simulink model "LKA_Real_Life_Reference_cs"  (Simulink 7.6)
settings: Solver=ode5  SolverMode=SingleTasking  StartTime=0.0  StopTime=inf  FixedStep=1/100  RelTol=1e-3
model workspace (MAT data in the model file):
  mbox_table : struct (value not decoded)
  model_closed = 0
  sim_status = 0
PreLoadFcn:
  | check_NUTS_defined;
  | 
  | assignin('base',['model_closed_' bdroot],false);
  | 
  | if exist('LDW_mask_sfun.mexw64')==3
  | delete LDW_mask_sfun.mexw64;
  | end
  | 
  | if exist('LDW_mask_sfun.mexw32') ==3
  | delete LDW_mask_sfun.mexw32;
  | end
  | 
  | switch version('-release')
  | case {'2010a', '2010b', '2011a'}
  | addpath('../includes_LKAS/includes_LKAS_2010a');
  | case {'2011b', '2012a', '2012b'}
  | addpath('../includes_LKAS/includes_LKAS_2011b');
  | otherwise
  | addpath('../includes_LKAS/includes_LKAS_2011b');
  | end
InitFcn:
  | PreScanExperimentInitFcn(bdroot)
StopFcn:
  | PreScanExperimentStopFcn(bdroot)
undefined symbols (not defined in the file): DiscIntNeverNeededParam, InitImageOutputDir, x_ini_VRML, y_ini_VRML, z_ini_VRML
libraries: PreScanSimulinkLibrary x84, simulink x28, PreScanUsefulBlocks x25, simulink_extras x23, dspsnks4 x2, simulink_need_slupdate x1
(part 1 of 33)
CloseFcn:
  | evalin('base',['clear model_closed_' bdroot]);
  | 
  | set(0, 'ShowHiddenHandles', 'on')
  | 
  | try
  |     close (PlotSpeed)
  | end
  | 
  | try
  |     close(findobj('Name','Filtered'))
  | end
  | 
  | try
  |     close(findobj('Name','Results'))
  | end
  | 
  | set(0, 'ShowHiddenHandles', 'off')
settings Model: Creator=PRESCAN  LastModifiedBy=buildbot
settings OptimizationCC: InitFltsAndDblsToZero=on
settings DebuggingCC: SaveWithParameterizedLinksMsg=none  SignalResolutionControl=TryResolveAllWithWarning  SolverPrmCheckMsg=none  MultiTaskDSMMsg=warning  MultiTaskCondExecSysMsg=none
settings SFSimCC: SimParseCustomCode=off
settings RTWCC: IncludeERTFirstTime=on
settings GRTTargetCC: TargetFcnLib=ansi_tfl_tmw.mat

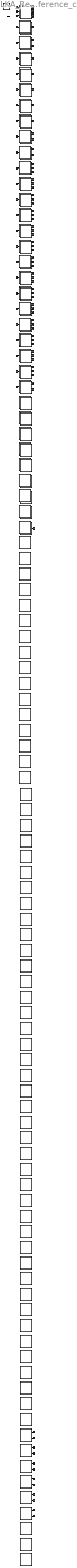
== system LKA_Real_Life_Reference_cs ==
  note: Demo Help
  note: Regenerate
  note: Record for Replay
  note: Open Useful Blocks
  note: PreScan Options
  note: Version:
  note: 7.4.0
  note: Author(s):
  note: N.A.
  note: Descriptive text:
  note: ID
      |
      | 1
      | 2
      | 483
      | 484
      | 485
      | 828
      | 830
      | 486
      | 838
      | 959
      | 992
      | 999
      | 1001
      | 1002
      | 1003
      | 1004
      | 1005
      | 1006
      | 1007
      | 1008
      | 1009
      | 1010
      | 1011
      | 1012
      | 1000
      | 639
      | 751
      | 757
      | 758
      | 759
      | 760
      | 761
      | 762
      | 763
      | 764
      | 765
      | 766
      | 767
      | 768
      | 769
      | 770
      | 771
      | 772
      | 773
      | 774
      | 775
      | 776
      | 777
      | 778
      | 779
      | 780
      | 781
      | 782
      | 783
      | 784
      | 785
      | 786
      | 787
      | 788
      | 789
      | 790
      | 791
      | 792
      | 793
      | 794
      | 795
      | 796
      | 797
      | 798
      | 799
      | 800
      | 801
      | 802
      | 803
      | 804
      | 805
      | 806
      | 807
      | 808
      | 809
      | 810
      | 811
      | 812
      | 813
      | 814
      | 815
      | 816
      | 817
      | 818
      | 819
      | 820
      | 115
      | 973
      | 985
      | 988
      | 993
      | 994
      | 1069
      | 1070
      | 1085
      | 1086
      | 1097
      | 1098
      | 1111
      | 1112
      | 1125
      | 1126
      | 1139
      | 1140
      | 1148
      | 1149
      | 1156
      | 1157
      | 1170
      | 1171
      | 1186
      | 1187
      | 1198
      | 1199
      | 1212
      | 1213
      | 1226
      | 1227
      | 1240
      | 1241
      | 1254
      | 1255
      | 1268
      | 1269
      | 1282
      | 1283
      | 1296
      | 1297
      | 1309
      | 1141
      | 1476
      | 1477
      | 1478
      | 1479
      | 1480
      | 1481
      | 1482
      | 1483
      | 1484
      | 1485
      | 1486
      | 1487
      | 1488
      | 1489
      | 1490
      | 1491
      | 1492
      | 1493
      | 1494
      | 1495
      | 1496
      | 1497
      | 1498
      | 1499
      | 1500
      | 1501
      | 1502
      | 1503
      | 1504
      | 1505
  note: OBU-Beacon ID
  note: TypeID
      |
      | 1
      | 5
      | 3
      | 3
      | 7
      | 3
      | 7
      | 3
      | 1
      | 1
      | 1
      | 1
      | 1
      | 1
      | 1
      | 1
      | 1
      | 1
      | 1
      | 1
      | 1
      | 1
      | 1
      | 1
      | 3
      | 15
      | 15
      | 15
      | 15
      | 15
      | 15
      | 15
      | 15
      | 15
      | 15
      | 15
      | 15
      | 15
      | 15
      | 15
      | 15
      | 15
      | 15
      | 15
      | 15
      | 15
      | 15
      | 15
      | 15
      | 15
      | 15
      | 15
      | 15
      | 15
      | 15
      | 15
      | 15
      | 15
      | 15
      | 15
      | 15
      | 15
      | 15
      | 15
      | 15
      | 15
      | 15
      | 15
      | 15
      | 15
      | 15
      | 15
      | 15
      | 15
      | 15
      | 15
      | 15
      | 15
      | 15
      | 15
      | 15
      | 15
      | 15
      | 15
      | 15
      | 15
      | 15
      | 15
      | 15
      | 15
      | 15
      | 15
      | 15
      | 15
      | 15
      | 15
      | 15
      | 15
      | 15
      | 15
      | 15
      | 15
      | 15
      | 15
      | 15
      | 15
      | 15
      | 15
      | 15
      | 15
      | 15
      | 15
      | 15
      | 15
      | 15
      | 15
      | 15
      | 15
      | 15
      | 15
      | 15
      | 15
      | 15
      | 15
      | 15
      | 15
      | 15
      | 15
      | 15
      | 15
      | 15
      | 15
      | 15
      | 15
      | 15
      | 15
      | 15
      | 15
      | 15
      | 15
      | 15
      | 15
      | 15
      | 15
      | 15
      | 15
      | 15
      | 15
      | 15
      | 15
      | 15
      | 15
      | 15
      | 15
      | 15
      | 15
      | 15
      | 15
      | 15
      | 15
      | 15
      | 15
      | 15
      | 15
      | 15
  note: TypeTag
      |
      | Car
      | Cal element
      | Truck\Bus
      | Truck\Bus
      | Unknown
      | Truck\Bus
      | Unknown
      | Truck\Bus
      | Car
      | Car
      | Car
      | Car
      | Car
      | Car
      | Car
      | Car
      | Car
      | Car
      | Car
      | Car
      | Car
      | Car
      | Car
      | Car
      | Truck\Bus
      | Anim traffic sign
      | Anim traffic sign
      | Anim traffic sign
      | Anim traffic sign
      | Anim traffic sign
      | Anim traffic sign
      | Anim traffic sign
      | Anim traffic sign
      | Anim traffic sign
      | Anim traffic sign
      | Anim traffic sign
      | Anim traffic sign
      | Anim traffic sign
      | Anim traffic sign
      | Anim traffic sign
      | Anim traffic sign
      | Anim traffic sign
      | Anim traffic sign
      | Anim traffic sign
      | Anim traffic sign
      | Anim traffic sign
      | Anim traffic sign
      | Anim traffic sign
      | Anim traffic sign
      | Anim traffic sign
      | Anim traffic sign
      | Anim traffic sign
      | Anim traffic sign
      | Anim traffic sign
      | Anim traffic sign
      | Anim traffic sign
      | Anim traffic sign
      | Anim traffic sign
      | Anim traffic sign
      | Anim traffic sign
      | Anim traffic sign
      | Anim traffic sign
      | Anim traffic sign
      | Anim traffic sign
      | Anim traffic sign
      | Anim traffic sign
      | Anim traffic sign
      | Anim traffic sign
      | Anim traffic sign
      | Anim traffic sign
      | Anim traffic sign
      | Anim traffic sign
      | Anim traffic sign
      | Anim traffic sign
      | Anim traffic sign
      | Anim traffic sign
      | Anim traffic sign
      | Anim traffic sign
      | Anim traffic sign
      | Anim traffic sign
      | Anim traffic sign
      | Anim traffic sign
      | Anim traffic sign
      | Anim traffic sign
      | Anim traffic sign
      | Anim traffic sign
      | Anim traffic sign
      | Anim traffic sign
      | Anim traffic sign
      | Anim traffic sign
      | Anim traffic sign
      | Anim traffic sign
      | Anim traffic sign
      | Anim traffic sign
      | Anim traffic sign
      | Anim traffic sign
      | Anim traffic sign
      | Anim traffic sign
      | Anim traffic sign
      | Anim traffic sign
      | Anim traffic sign
      | Anim traffic sign
      | Anim traffic sign
      | Anim traffic sign
      | Anim traffic sign
      | Anim traffic sign
      | Anim traffic sign
      | Anim traffic sign
      | Anim traffic sign
      | Anim traffic sign
      | Anim traffic sign
      | Anim traffic sign
      | Anim traffic sign
      | Anim traffic sign
      | Anim traffic sign
      | Anim traffic sign
      | Anim traffic sign
      | Anim traffic sign
      | Anim traffic sign
      | Anim traffic sign
      | Anim traffic sign
      | Anim traffic sign
      | Anim traffic sign
      | Anim traffic sign
      | Anim traffic sign
      | Anim traffic sign
      | Anim traffic sign
      | Anim traffic sign
      | Anim traffic sign
      | Anim traffic sign
      | Anim traffic sign
      | Anim traffic sign
      | Anim traffic sign
      | Anim traffic sign
      | Anim traffic sign
      | Anim traffic sign
      | Anim traffic sign
      | Anim traffic sign
      | Anim traffic sign
      | Anim traffic sign
      | Anim traffic sign
      | Anim traffic sign
      | Anim traffic sign
      | Anim traffic sign
      | Anim traffic sign
      | Anim traffic sign
      | Anim traffic sign
      | Anim traffic sign
      | Anim traffic sign
      | Anim traffic sign
      | Anim traffic sign
      | Anim traffic sign
      | Anim traffic sign
      | Anim traffic sign
      | Anim traffic sign
      | Anim traffic sign
      | Anim traffic sign
      | Anim traffic sign
      | Anim traffic sign
      | Anim traffic sign
      | Anim traffic sign
      | Anim traffic sign
      | Anim traffic sign
      | Anim traffic sign
      | Anim traffic sign
  note: SD
      |
      | Y
      | Y
      | Y
      | Y
      | Y
      | Y
      | Y
      | Y
      | Y
      | Y
      | Y
      | Y
      | Y
      | Y
      | Y
      | Y
      | Y
      | Y
      | Y
      | Y
      | Y
      | Y
      | Y
      | Y
      | Y
      | N
      | N
      | N
      | N
      | N
      | N
      | N
      | N
      | N
      | N
      | N
      | N
      | N
      | N
      | N
      | N
      | N
      | N
      | N
      | N
      | N
      | N
      | N
      | N
      | N
      | N
      | N
      | N
      | N
      | N
      | N
      | N
      | N
      | N
      | N
      | N
      | N
      | N
      | N
      | N
      | N
      | N
      | N
      | N
      | N
      | N
      | N
      | N
      | N
      | N
      | N
      | N
      | N
      | N
      | N
      | N
      | N
      | N
      | N
      | N
      | N
      | N
      | N
      | N
      | N
      | N
      | Y
      | Y
      | Y
      | Y
      | Y
      | Y
      | N
      | N
      | N
      | N
      | N
      | N
      | N
      | N
      | N
      | N
      | N
      | N
      | N
      | N
      | N
      | N
      | N
      | N
      | N
      | N
      | N
      | N
      | N
      | N
      | N
      | N
      | N
      | N
      | N
      | N
      | N
      | N
      | N
      | N
      | N
      | N
      | Y
      | Y
      | Y
      | Y
      | Y
      | Y
      | Y
      | Y
      | Y
      | Y
      | Y
      | Y
      | Y
      | Y
      | Y
      | Y
      | Y
      | Y
      | Y
      | Y
      | Y
      | Y
      | Y
      | Y
      | Y
      | Y
      | Y
      | Y
      | Y
      | Y
      | Y
      | Y
  note: CD
      |
      | N
      | N
      | N
      | N
      | N
      | N
      | N
      | N
      | N
      | N
      | N
      | N
      | N
      | N
      | N
      | N
      | N
      | N
      | N
      | N
      | N
      | N
      | N
      | N
      | N
      | N
      | N
      | N
      | N
      | N
      | N
      | N
      | N
      | N
      | N
      | N
      | N
      | N
      | N
      | N
      | N
      | N
      | N
      | N
      | N
      | N
      | N
      | N
      | N
      | N
      | N
      | N
      | N
      | N
      | N
      | N
      | N
      | N
      | N
      | N
      | N
      | N
      | N
      | N
      | N
      | N
      | N
      | N
      | N
      | N
      | N
      | N
      | N
      | N
      | N
      | N
      | N
      | N
      | N
      | N
      | N
      | N
      | N
      | N
      | N
      | N
      | N
      | N
      | N
      | N
      | N
      | N
      | N
      | N
      | N
      | N
      | N
      | N
      | N
      | N
      | N
      | N
      | N
      | N
      | N
      | N
      | N
      | N
      | N
      | N
      | N
      | N
      | N
      | N
      | N
      | N
      | N
      | N
      | N
      | N
      | N
      | N
      | N
      | N
      | N
      | N
      | N
      | N
      | N
      | N
      | N
      | N
      | N
      | N
      | N
      | N
      | N
      | N
      | N
      | N
      | N
      | N
      | N
      | N
      | N
      | N
      | N
      | N
      | N
      | N
      | N
      | N
      | N
      | N
      | N
      | N
      | N
      | N
      | N
      | N
      | N
      | N
      | N
      | N
      | N
  note: Name
      |
      | Audi_A8_1
      | Sphere_1
      | Nissan_Cabstar_1
      | Actros_1860_1
      | ContainerTrailer_DoubleAxle_1
      | Actros_1860_2
      | ContainerTrailer_DoubleAxle_2
      | Actros_2541_1
      | Toyota_Previa_1
      | Mazda_RX8_1
      | Mazda_RX8_2
      | Ford_Fiesta_1
      | BMW_Z3_1
      | Audi_A8_2
      | Citroen_C3_1
      | Citroen_C3_2
      | Toyota_Yaris_1
      | Fiat_Bravo_1
      | Citroen_C3_3
      | Toyota_Previa_2
      | Mazda_RX8_3
      | Fiat_Bravo_2
      | BMW_Z3_2
      | Citroen_C3_4
      | Actros_2541_2
      | Lightpost_1
      | Lightpost_2
      | Lightpost_3
      | Lightpost_4
      | Lightpost_5
      | Lightpost_6
      | Lightpost_7
      | Lightpost_8
      | Lightpost_9
      | Lightpost_10
      | Lightpost_11
      | Lightpost_12
      | Lightpost_13
      | Lightpost_14
      | Lightpost_15
      | Lightpost_16
      | Lightpost_17
      | Lightpost_18
      | Lightpost_19
      | Lightpost_20
      | Lightpost_21
      | Lightpost_22
      | Lightpost_23
      | Lightpost_24
      | Lightpost_25
      | Lightpost_26
      | Lightpost_27
      | Lightpost_28
      | Lightpost_29
      | Lightpost_30
      | Lightpost_31
      | Lightpost_32
      | Lightpost_33
      | Lightpost_34
      | Lightpost_35
      | Lightpost_36
      | Lightpost_37
      | Lightpost_38
      | Lightpost_39
      | Lightpost_40
      | Lightpost_41
      | Lightpost_42
      | Lightpost_43
      | Lightpost_44
      | Lightpost_45
      | Lightpost_46
      | Lightpost_47
      | Lightpost_48
      | Lightpost_49
      | Lightpost_50
      | Lightpost_51
      | Lightpost_52
      | Lightpost_53
      | Lightpost_54
      | Lightpost_55
      | Lightpost_56
      | Lightpost_57
      | Lightpost_58
      | Lightpost_59
      | Lightpost_60
      | Lightpost_61
      | Lightpost_62
      | Lightpost_63
      | Lightpost_64
      | Lightpost_65
      | Lightpost_66
      | HighwayOverhang_1
      | HighwayOverhang_2
      | HighwayOverhang_3
      | HighwayOverhang_4
      | HighwayOverhang_5
      | HighwayOverhang_6
      | Lightpost_67
      | Lightpost_68
      | Lightpost_71
      | Lightpost_72
      | Lightpost_69
      | Lightpost_70
      | Lightpost_73
      | Lightpost_74
      | Lightpost_75
      | Lightpost_76
      | Lightpost_77
      | Lightpost_78
      | Lightpost_79
      | Lightpost_80
      | Lightpost_81
      | Lightpost_82
      | Lightpost_83
      | Lightpost_84
      | Lightpost_87
      | Lightpost_88
      | Lightpost_85
      | Lightpost_86
      | Lightpost_89
      | Lightpost_90
      | Lightpost_91
      | Lightpost_92
      | Lightpost_93
      | Lightpost_94
      | Lightpost_95
      | Lightpost_96
      | Lightpost_97
      | Lightpost_98
      | Lightpost_99
      | Lightpost_100
      | Lightpost_101
      | Lightpost_102
      | InformationBoard_1
      | InformationBoard_2
      | InformationBoard_3
      | InformationBoard_4
      | InformationBoard_5
      | InformationBoard_6
      | InformationBoard_7
      | InformationBoard_8
      | InformationBoard_9
      | InformationBoard_10
      | InformationBoard_11
      | InformationBoard_12
      | InformationBoard_13
      | InformationBoard_14
      | InformationBoard_15
      | InformationBoard_16
      | InformationBoard_17
      | InformationBoard_18
      | InformationBoard_19
      | InformationBoard_20
      | InformationBoard_21
      | InformationBoard_22
      | InformationBoard_23
      | InformationBoard_24
      | InformationBoard_25
      | InformationBoard_26
      | InformationBoard_27
      | InformationBoard_28
      | InformationBoard_29
      | InformationBoard_30
      | InformationBoard_31
      | HighwayOverhang_7
  note: Num ID OBU-Beacon ID Type ID Type Tag SD CD Name
  Actros_1860_1 : Subsystem  Tag=WorldObjectSubSystem  InitFcn=UniqueId = '1580';
      mask Display: image(imread('Actros_1860.png'));color('white');text(0,0,' ID=484\n')
  Actros_1860_2 : Subsystem  Tag=WorldObjectSubSystem  InitFcn=UniqueId = '1584';
      mask Display: image(imread('Actros_1860.png'));color('white');text(0,0,' ID=828\n')
  Actros_2541_1 : Subsystem  Tag=WorldObjectSubSystem  InitFcn=UniqueId = '1588';
      mask Display: image(imread('Actros_2541.png'));color('white');text(0,0,' ID=486\n')
  Actros_2541_2 : Subsystem  Tag=WorldObjectSubSystem  InitFcn=UniqueId = '2575';
      mask Display: image(imread('Actros_2541.png'));color('white');text(0,0,' ID=1000\n')
  Actuators : DataStoreMemory  Tag=Actuators  DataStoreName=SimCoreActuators  InitialValue=zeros(2639,1)
      settings: DeleteFcn=PreScanBlockDeleteFcn;
  Audi_A8_1 : Subsystem  Tag=WorldObjectSubSystem  InitFcn=UniqueId = '253';
      mask Display: image(imread('Audi_A8.jpg'));color('white');text(0,0,' ID=1\n')
  Audi_A8_2 : Subsystem  Tag=WorldObjectSubSystem  InitFcn=UniqueId = '1951';
      mask Display: image(imread('Audi_A8.jpg'));color('white');text(0,0,' ID=1002\n')
  BMW_Z3_1 : Subsystem  Tag=WorldObjectSubSystem  InitFcn=UniqueId = '1948';
      mask Display: image(imread('BMW_Z3.jpg'));color('white');text(0,0,' ID=1001\n')
  BMW_Z3_2 : Subsystem  Tag=WorldObjectSubSystem  InitFcn=UniqueId = '2024';
      mask Display: image(imread('BMW_Z3.jpg'));color('white');text(0,0,' ID=1011\n')
  CameraSensor_2 : Subsystem  Tag=CameraSensor  Priority=3
      settings: DeleteFcn=PreScanBlockDeleteFcn;
      mask Type: PreScan Source Block: Camera Sensor
      mask Description: The Camera Sensor provides a color or monochrome image.
      mask Help: eval('openHelp(457)')
      mask Display: image(imread('CameraSensor.png'))
  Citroen_C3_1 : Subsystem  Tag=WorldObjectSubSystem  InitFcn=UniqueId = '1955';
      mask Display: image(imread('Citroen_C3.jpg'));color('white');text(0,0,' ID=1003\n')
  Citroen_C3_2 : Subsystem  Tag=WorldObjectSubSystem  InitFcn=UniqueId = '1958';
      mask Display: image(imread('Citroen_C3.jpg'));color('white');text(0,0,' ID=1004\n')
  Citroen_C3_3 : Subsystem  Tag=WorldObjectSubSystem  InitFcn=UniqueId = '1967';
      mask Display: image(imread('Citroen_C3.jpg'));color('white');text(0,0,' ID=1007\n')
  Citroen_C3_4 : Subsystem  Tag=WorldObjectSubSystem  InitFcn=UniqueId = '2027';
      mask Display: image(imread('Citroen_C3.jpg'));color('white');text(0,0,' ID=1012\n')
  ContainerTrailer_DoubleAxle_1 : Subsystem  Tag=WorldObjectSubSystem  InitFcn=UniqueId = '1582';
      mask Display: image(imread('ContainerTrailer_DoubleAxle.jpg'));color('white');text(0,0,' ID=485\n')
  ContainerTrailer_DoubleAxle_2 : Subsystem  Tag=WorldObjectSubSystem  InitFcn=UniqueId = '1586';
      mask Display: image(imread('ContainerTrailer_DoubleAxle.jpg'));color('white');text(0,0,' ID=830\n')
  Fiat_Bravo_1 : Subsystem  Tag=WorldObjectSubSystem  InitFcn=UniqueId = '1964';
      mask Display: image(imread('Fiat_Bravo.jpg'));color('white');text(0,0,' ID=1006\n')
  Fiat_Bravo_2 : Subsystem  Tag=WorldObjectSubSystem  InitFcn=UniqueId = '2021';
      mask Display: image(imread('Fiat_Bravo.jpg'));color('white');text(0,0,' ID=1010\n')
  Ford_Fiesta_1 : Subsystem  Tag=WorldObjectSubSystem  InitFcn=UniqueId = '1934';
      mask Display: image(imread('Ford_Fiesta.jpg'));color('white');text(0,0,' ID=999\n')
  GetSensors : Subsystem  Tag=GetSensors  Priority=2  mask(PreScan Simulation Core Block){NrOfSensors=200, TimeStep=1/100}
      mask prompts: NrOfSensors "Number of sensor values"; TimeStep "Timestep [s]"
      mask help:
      | Please do not remove this block from the PreScan compilation sheet,
      | as it contains essential configuration parameters for a PreScan simulation.
      settings: DeleteFcn=PreScanBlockDeleteFcn;
  HighwayOverhang_1 : Subsystem  Tag=WorldObjectSubSystem  InitFcn=UniqueId = '1876';
      mask Display: image(imread('HighwayOverhang.jpg'));color('white');text(0,0,' ID=115\n')
  HighwayOverhang_2 : Subsystem  Tag=WorldObjectSubSystem  InitFcn=UniqueId = '1877';
      mask Display: image(imread('HighwayOverhang.jpg'));color('white');text(0,0,' ID=973\n')
  HighwayOverhang_3 : Subsystem  Tag=WorldObjectSubSystem  InitFcn=UniqueId = '1890';
      mask Display: image(imread('HighwayOverhang.jpg'));color('white');text(0,0,' ID=985\n')
  HighwayOverhang_4 : Subsystem  Tag=WorldObjectSubSystem  InitFcn=UniqueId = '1898';
      mask Display: image(imread('HighwayOverhang.jpg'));color('white');text(0,0,' ID=988\n')
  HighwayOverhang_5 : Subsystem  Tag=WorldObjectSubSystem  InitFcn=UniqueId = '1909';
      mask Display: image(imread('HighwayOverhang.jpg'));color('white');text(0,0,' ID=993\n')
  HighwayOverhang_6 : Subsystem  Tag=WorldObjectSubSystem  InitFcn=UniqueId = '1914';
      mask Display: image(imread('HighwayOverhang.jpg'));color('white');text(0,0,' ID=994\n')
  HighwayOverhang_7 : Subsystem  Tag=WorldObjectSubSystem  InitFcn=UniqueId = '2612';
      mask Display: image(imread('HighwayOverhang.jpg'));color('white');text(0,0,' ID=1505\n')
  LaneMarkerSensor_1 : Subsystem  Tag=LaneMarkerSensor  Priority=3
      settings: DeleteFcn=PreScanBlockDeleteFcn;
      mask Type: PreScan Source Block: Lane Marker Sensor
      mask Description: The Lane Marker Sensor provides information relating the sensor to the lane markers on the road.
      mask Help: eval('openHelp(457)')
      mask Display: image(imread('LaneMarkerSensorSL.png'))
  Lightpost_1 : Subsystem  Tag=WorldObjectSubSystem  InitFcn=UniqueId = '1495';
      mask Display: image(imread('Lightpost.jpg'));color('white');text(0,0,' ID=639\n')
  Lightpost_10 : Subsystem  Tag=WorldObjectSubSystem  InitFcn=UniqueId = '1504';
      mask Display: image(imread('Lightpost.jpg'));color('white');text(0,0,' ID=764\n')
  Lightpost_100 : Subsystem  Tag=WorldObjectSubSystem  InitFcn=UniqueId = '2375';
      mask Display: image(imread('Lightpost.jpg'));color('white');text(0,0,' ID=1283\n')
  Lightpost_101 : Subsystem  Tag=WorldObjectSubSystem  InitFcn=UniqueId = '2390';
      mask Display: image(imread('Lightpost.jpg'));color('white');text(0,0,' ID=1296\n')
  Lightpost_102 : Subsystem  Tag=WorldObjectSubSystem  InitFcn=UniqueId = '2391';
      mask Display: image(imread('Lightpost.jpg'));color('white');text(0,0,' ID=1297\n')
  Lightpost_11 : Subsystem  Tag=WorldObjectSubSystem  InitFcn=UniqueId = '1505';
      mask Display: image(imread('Lightpost.jpg'));color('white');text(0,0,' ID=765\n')
  Lightpost_12 : Subsystem  Tag=WorldObjectSubSystem  InitFcn=UniqueId = '1506';
      mask Display: image(imread('Lightpost.jpg'));color('white');text(0,0,' ID=766\n')
  Lightpost_13 : Subsystem  Tag=WorldObjectSubSystem  InitFcn=UniqueId = '1507';
      mask Display: image(imread('Lightpost.jpg'));color('white');text(0,0,' ID=767\n')
  Lightpost_14 : Subsystem  Tag=WorldObjectSubSystem  InitFcn=UniqueId = '1508';
      mask Display: image(imread('Lightpost.jpg'));color('white');text(0,0,' ID=768\n')
  Lightpost_15 : Subsystem  Tag=WorldObjectSubSystem  InitFcn=UniqueId = '1509';
      mask Display: image(imread('Lightpost.jpg'));color('white');text(0,0,' ID=769\n')
  Lightpost_16 : Subsystem  Tag=WorldObjectSubSystem  InitFcn=UniqueId = '1510';
      mask Display: image(imread('Lightpost.jpg'));color('white');text(0,0,' ID=770\n')
  Lightpost_17 : Subsystem  Tag=WorldObjectSubSystem  InitFcn=UniqueId = '1511';
      mask Display: image(imread('Lightpost.jpg'));color('white');text(0,0,' ID=771\n')
  Lightpost_18 : Subsystem  Tag=WorldObjectSubSystem  InitFcn=UniqueId = '1512';
      mask Display: image(imread('Lightpost.jpg'));color('white');text(0,0,' ID=772\n')
  Lightpost_19 : Subsystem  Tag=WorldObjectSubSystem  InitFcn=UniqueId = '1513';
      mask Display: image(imread('Lightpost.jpg'));color('white');text(0,0,' ID=773\n')
  Lightpost_2 : Subsystem  Tag=WorldObjectSubSystem  InitFcn=UniqueId = '1496';
      mask Display: image(imread('Lightpost.jpg'));color('white');text(0,0,' ID=751\n')
  Lightpost_20 : Subsystem  Tag=WorldObjectSubSystem  InitFcn=UniqueId = '1514';
      mask Display: image(imread('Lightpost.jpg'));color('white');text(0,0,' ID=774\n')
  Lightpost_21 : Subsystem  Tag=WorldObjectSubSystem  InitFcn=UniqueId = '1515';
      mask Display: image(imread('Lightpost.jpg'));color('white');text(0,0,' ID=775\n')
  Lightpost_22 : Subsystem  Tag=WorldObjectSubSystem  InitFcn=UniqueId = '1516';
      mask Display: image(imread('Lightpost.jpg'));color('white');text(0,0,' ID=776\n')
  Lightpost_23 : Subsystem  Tag=WorldObjectSubSystem  InitFcn=UniqueId = '1517';
      mask Display: image(imread('Lightpost.jpg'));color('white');text(0,0,' ID=777\n')
  Lightpost_24 : Subsystem  Tag=WorldObjectSubSystem  InitFcn=UniqueId = '1518';
      mask Display: image(imread('Lightpost.jpg'));color('white');text(0,0,' ID=778\n')
  Lightpost_25 : Subsystem  Tag=WorldObjectSubSystem  InitFcn=UniqueId = '1519';
      mask Display: image(imread('Lightpost.jpg'));color('white');text(0,0,' ID=779\n')
  Lightpost_26 : Subsystem  Tag=WorldObjectSubSystem  InitFcn=UniqueId = '1520';
      mask Display: image(imread('Lightpost.jpg'));color('white');text(0,0,' ID=780\n')
  Lightpost_27 : Subsystem  Tag=WorldObjectSubSystem  InitFcn=UniqueId = '1521';
      mask Display: image(imread('Lightpost.jpg'));color('white');text(0,0,' ID=781\n')
  Lightpost_28 : Subsystem  Tag=WorldObjectSubSystem  InitFcn=UniqueId = '1522';
      mask Display: image(imread('Lightpost.jpg'));color('white');text(0,0,' ID=782\n')
  Lightpost_29 : Subsystem  Tag=WorldObjectSubSystem  InitFcn=UniqueId = '1523';
      mask Display: image(imread('Lightpost.jpg'));color('white');text(0,0,' ID=783\n')
  Lightpost_3 : Subsystem  Tag=WorldObjectSubSystem  InitFcn=UniqueId = '1497';
      mask Display: image(imread('Lightpost.jpg'));color('white');text(0,0,' ID=757\n')
  Lightpost_30 : Subsystem  Tag=WorldObjectSubSystem  InitFcn=UniqueId = '1524';
      mask Display: image(imread('Lightpost.jpg'));color('white');text(0,0,' ID=784\n')
  Lightpost_31 : Subsystem  Tag=WorldObjectSubSystem  InitFcn=UniqueId = '1525';
      mask Display: image(imread('Lightpost.jpg'));color('white');text(0,0,' ID=785\n')
  Lightpost_32 : Subsystem  Tag=WorldObjectSubSystem  InitFcn=UniqueId = '1526';
      mask Display: image(imread('Lightpost.jpg'));color('white');text(0,0,' ID=786\n')
  Lightpost_33 : Subsystem  Tag=WorldObjectSubSystem  InitFcn=UniqueId = '1527';
      mask Display: image(imread('Lightpost.jpg'));color('white');text(0,0,' ID=787\n')
  Lightpost_34 : Subsystem  Tag=WorldObjectSubSystem  InitFcn=UniqueId = '1528';
      mask Display: image(imread('Lightpost.jpg'));color('white');text(0,0,' ID=788\n')
  Lightpost_35 : Subsystem  Tag=WorldObjectSubSystem  InitFcn=UniqueId = '1529';
      mask Display: image(imread('Lightpost.jpg'));color('white');text(0,0,' ID=789\n')
  Lightpost_36 : Subsystem  Tag=WorldObjectSubSystem  InitFcn=UniqueId = '1530';
      mask Display: image(imread('Lightpost.jpg'));color('white');text(0,0,' ID=790\n')
  Lightpost_37 : Subsystem  Tag=WorldObjectSubSystem  InitFcn=UniqueId = '1531';
      mask Display: image(imread('Lightpost.jpg'));color('white');text(0,0,' ID=791\n')
  Lightpost_38 : Subsystem  Tag=WorldObjectSubSystem  InitFcn=UniqueId = '1532';
      mask Display: image(imread('Lightpost.jpg'));color('white');text(0,0,' ID=792\n')
  Lightpost_39 : Subsystem  Tag=WorldObjectSubSystem  InitFcn=UniqueId = '1533';
      mask Display: image(imread('Lightpost.jpg'));color('white');text(0,0,' ID=793\n')
  Lightpost_4 : Subsystem  Tag=WorldObjectSubSystem  InitFcn=UniqueId = '1498';
      mask Display: image(imread('Lightpost.jpg'));color('white');text(0,0,' ID=758\n')
  Lightpost_40 : Subsystem  Tag=WorldObjectSubSystem  InitFcn=UniqueId = '1534';
      mask Display: image(imread('Lightpost.jpg'));color('white');text(0,0,' ID=794\n')
  Lightpost_41 : Subsystem  Tag=WorldObjectSubSystem  InitFcn=UniqueId = '1535';
      mask Display: image(imread('Lightpost.jpg'));color('white');text(0,0,' ID=795\n')
  Lightpost_42 : Subsystem  Tag=WorldObjectSubSystem  InitFcn=UniqueId = '1536';
      mask Display: image(imread('Lightpost.jpg'));color('white');text(0,0,' ID=796\n')
  Lightpost_43 : Subsystem  Tag=WorldObjectSubSystem  InitFcn=UniqueId = '1537';
      mask Display: image(imread('Lightpost.jpg'));color('white');text(0,0,' ID=797\n')
  Lightpost_44 : Subsystem  Tag=WorldObjectSubSystem  InitFcn=UniqueId = '1538';
      mask Display: image(imread('Lightpost.jpg'));color('white');text(0,0,' ID=798\n')
  Lightpost_45 : Subsystem  Tag=WorldObjectSubSystem  InitFcn=UniqueId = '1539';
      mask Display: image(imread('Lightpost.jpg'));color('white');text(0,0,' ID=799\n')
  Lightpost_46 : Subsystem  Tag=WorldObjectSubSystem  InitFcn=UniqueId = '1540';
      mask Display: image(imread('Lightpost.jpg'));color('white');text(0,0,' ID=800\n')
  Lightpost_47 : Subsystem  Tag=WorldObjectSubSystem  InitFcn=UniqueId = '1541';
      mask Display: image(imread('Lightpost.jpg'));color('white');text(0,0,' ID=801\n')
  Lightpost_48 : Subsystem  Tag=WorldObjectSubSystem  InitFcn=UniqueId = '1542';
      mask Display: image(imread('Lightpost.jpg'));color('white');text(0,0,' ID=802\n')
  Lightpost_49 : Subsystem  Tag=WorldObjectSubSystem  InitFcn=UniqueId = '1543';
      mask Display: image(imread('Lightpost.jpg'));color('white');text(0,0,' ID=803\n')
  Lightpost_5 : Subsystem  Tag=WorldObjectSubSystem  InitFcn=UniqueId = '1499';
      mask Display: image(imread('Lightpost.jpg'));color('white');text(0,0,' ID=759\n')
  Lightpost_50 : Subsystem  Tag=WorldObjectSubSystem  InitFcn=UniqueId = '1544';
      mask Display: image(imread('Lightpost.jpg'));color('white');text(0,0,' ID=804\n')
  Lightpost_51 : Subsystem  Tag=WorldObjectSubSystem  InitFcn=UniqueId = '1545';
      mask Display: image(imread('Lightpost.jpg'));color('white');text(0,0,' ID=805\n')
  Lightpost_52 : Subsystem  Tag=WorldObjectSubSystem  InitFcn=UniqueId = '1546';
      mask Display: image(imread('Lightpost.jpg'));color('white');text(0,0,' ID=806\n')
  Lightpost_53 : Subsystem  Tag=WorldObjectSubSystem  InitFcn=UniqueId = '1547';
      mask Display: image(imread('Lightpost.jpg'));color('white');text(0,0,' ID=807\n')
  Lightpost_54 : Subsystem  Tag=WorldObjectSubSystem  InitFcn=UniqueId = '1548';
      mask Display: image(imread('Lightpost.jpg'));color('white');text(0,0,' ID=808\n')
  Lightpost_55 : Subsystem  Tag=WorldObjectSubSystem  InitFcn=UniqueId = '1549';
      mask Display: image(imread('Lightpost.jpg'));color('white');text(0,0,' ID=809\n')
  Lightpost_56 : Subsystem  Tag=WorldObjectSubSystem  InitFcn=UniqueId = '1550';
      mask Display: image(imread('Lightpost.jpg'));color('white');text(0,0,' ID=810\n')
  Lightpost_57 : Subsystem  Tag=WorldObjectSubSystem  InitFcn=UniqueId = '1551';
      mask Display: image(imread('Lightpost.jpg'));color('white');text(0,0,' ID=811\n')
  Lightpost_58 : Subsystem  Tag=WorldObjectSubSystem  InitFcn=UniqueId = '1552';
      mask Display: image(imread('Lightpost.jpg'));color('white');text(0,0,' ID=812\n')
  Lightpost_59 : Subsystem  Tag=WorldObjectSubSystem  InitFcn=UniqueId = '1553';
      mask Display: image(imread('Lightpost.jpg'));color('white');text(0,0,' ID=813\n')
  Lightpost_6 : Subsystem  Tag=WorldObjectSubSystem  InitFcn=UniqueId = '1500';
      mask Display: image(imread('Lightpost.jpg'));color('white');text(0,0,' ID=760\n')
  Lightpost_60 : Subsystem  Tag=WorldObjectSubSystem  InitFcn=UniqueId = '1554';
      mask Display: image(imread('Lightpost.jpg'));color('white');text(0,0,' ID=814\n')
  Lightpost_61 : Subsystem  Tag=WorldObjectSubSystem  InitFcn=UniqueId = '1555';
      mask Display: image(imread('Lightpost.jpg'));color('white');text(0,0,' ID=815\n')
  Lightpost_62 : Subsystem  Tag=WorldObjectSubSystem  InitFcn=UniqueId = '1556';
      mask Display: image(imread('Lightpost.jpg'));color('white');text(0,0,' ID=816\n')
  Lightpost_63 : Subsystem  Tag=WorldObjectSubSystem  InitFcn=UniqueId = '1557';
      mask Display: image(imread('Lightpost.jpg'));color('white');text(0,0,' ID=817\n')
  Lightpost_64 : Subsystem  Tag=WorldObjectSubSystem  InitFcn=UniqueId = '1558';
      mask Display: image(imread('Lightpost.jpg'));color('white');text(0,0,' ID=818\n')
  Lightpost_65 : Subsystem  Tag=WorldObjectSubSystem  InitFcn=UniqueId = '1559';
      mask Display: image(imread('Lightpost.jpg'));color('white');text(0,0,' ID=819\n')
  Lightpost_66 : Subsystem  Tag=WorldObjectSubSystem  InitFcn=UniqueId = '1560';
      mask Display: image(imread('Lightpost.jpg'));color('white');text(0,0,' ID=820\n')
  Lightpost_67 : Subsystem  Tag=WorldObjectSubSystem  InitFcn=UniqueId = '2124';
      mask Display: image(imread('Lightpost.jpg'));color('white');text(0,0,' ID=1069\n')
  Lightpost_68 : Subsystem  Tag=WorldObjectSubSystem  InitFcn=UniqueId = '2125';
      mask Display: image(imread('Lightpost.jpg'));color('white');text(0,0,' ID=1070\n')
  Lightpost_69 : Subsystem  Tag=WorldObjectSubSystem  InitFcn=UniqueId = '2156';
      mask Display: image(imread('Lightpost.jpg'));color('white');text(0,0,' ID=1097\n')
  Lightpost_7 : Subsystem  Tag=WorldObjectSubSystem  InitFcn=UniqueId = '1501';
      mask Display: image(imread('Lightpost.jpg'));color('white');text(0,0,' ID=761\n')
  Lightpost_70 : Subsystem  Tag=WorldObjectSubSystem  InitFcn=UniqueId = '2157';
      mask Display: image(imread('Lightpost.jpg'));color('white');text(0,0,' ID=1098\n')
  Lightpost_71 : Subsystem  Tag=WorldObjectSubSystem  InitFcn=UniqueId = '2140';
      mask Display: image(imread('Lightpost.jpg'));color('white');text(0,0,' ID=1085\n')
  Lightpost_72 : Subsystem  Tag=WorldObjectSubSystem  InitFcn=UniqueId = '2141';
      mask Display: image(imread('Lightpost.jpg'));color('white');text(0,0,' ID=1086\n')
  Lightpost_73 : Subsystem  Tag=WorldObjectSubSystem  InitFcn=UniqueId = '2172';
      mask Display: image(imread('Lightpost.jpg'));color('white');text(0,0,' ID=1111\n')
  Lightpost_74 : Subsystem  Tag=WorldObjectSubSystem  InitFcn=UniqueId = '2173';
      mask Display: image(imread('Lightpost.jpg'));color('white');text(0,0,' ID=1112\n')
  Lightpost_75 : Subsystem  Tag=WorldObjectSubSystem  InitFcn=UniqueId = '2188';
      mask Display: image(imread('Lightpost.jpg'));color('white');text(0,0,' ID=1125\n')
  Lightpost_76 : Subsystem  Tag=WorldObjectSubSystem  InitFcn=UniqueId = '2189';
      mask Display: image(imread('Lightpost.jpg'));color('white');text(0,0,' ID=1126\n')
  Lightpost_77 : Subsystem  Tag=WorldObjectSubSystem  InitFcn=UniqueId = '2204';
      mask Display: image(imread('Lightpost.jpg'));color('white');text(0,0,' ID=1139\n')
  Lightpost_78 : Subsystem  Tag=WorldObjectSubSystem  InitFcn=UniqueId = '2205';
      mask Display: image(imread('Lightpost.jpg'));color('white');text(0,0,' ID=1140\n')
  Lightpost_79 : Subsystem  Tag=WorldObjectSubSystem  InitFcn=UniqueId = '2221';
      mask Display: image(imread('Lightpost.jpg'));color('white');text(0,0,' ID=1148\n')
  Lightpost_8 : Subsystem  Tag=WorldObjectSubSystem  InitFcn=UniqueId = '1502';
      mask Display: image(imread('Lightpost.jpg'));color('white');text(0,0,' ID=762\n')
  Lightpost_80 : Subsystem  Tag=WorldObjectSubSystem  InitFcn=UniqueId = '2222';
      mask Display: image(imread('Lightpost.jpg'));color('white');text(0,0,' ID=1149\n')
  Lightpost_81 : Subsystem  Tag=WorldObjectSubSystem  InitFcn=UniqueId = '2229';
      mask Display: image(imread('Lightpost.jpg'));color('white');text(0,0,' ID=1156\n')
  Lightpost_82 : Subsystem  Tag=WorldObjectSubSystem  InitFcn=UniqueId = '2230';
      mask Display: image(imread('Lightpost.jpg'));color('white');text(0,0,' ID=1157\n')
  Lightpost_83 : Subsystem  Tag=WorldObjectSubSystem  InitFcn=UniqueId = '2245';
      mask Display: image(imread('Lightpost.jpg'));color('white');text(0,0,' ID=1170\n')
  Lightpost_84 : Subsystem  Tag=WorldObjectSubSystem  InitFcn=UniqueId = '2246';
      mask Display: image(imread('Lightpost.jpg'));color('white');text(0,0,' ID=1171\n')
  Lightpost_85 : Subsystem  Tag=WorldObjectSubSystem  InitFcn=UniqueId = '2278';
      mask Display: image(imread('Lightpost.jpg'));color('white');text(0,0,' ID=1198\n')
  Lightpost_86 : Subsystem  Tag=WorldObjectSubSystem  InitFcn=UniqueId = '2279';
      mask Display: image(imread('Lightpost.jpg'));color('white');text(0,0,' ID=1199\n')
  Lightpost_87 : Subsystem  Tag=WorldObjectSubSystem  InitFcn=UniqueId = '2261';
      mask Display: image(imread('Lightpost.jpg'));color('white');text(0,0,' ID=1186\n')
  Lightpost_88 : Subsystem  Tag=WorldObjectSubSystem  InitFcn=UniqueId = '2262';
      mask Display: image(imread('Lightpost.jpg'));color('white');text(0,0,' ID=1187\n')
  Lightpost_89 : Subsystem  Tag=WorldObjectSubSystem  InitFcn=UniqueId = '2294';
      mask Display: image(imread('Lightpost.jpg'));color('white');text(0,0,' ID=1212\n')
  Lightpost_9 : Subsystem  Tag=WorldObjectSubSystem  InitFcn=UniqueId = '1503';
      mask Display: image(imread('Lightpost.jpg'));color('white');text(0,0,' ID=763\n')
  Lightpost_90 : Subsystem  Tag=WorldObjectSubSystem  InitFcn=UniqueId = '2295';
      mask Display: image(imread('Lightpost.jpg'));color('white');text(0,0,' ID=1213\n')
  Lightpost_91 : Subsystem  Tag=WorldObjectSubSystem  InitFcn=UniqueId = '2310';
      mask Display: image(imread('Lightpost.jpg'));color('white');text(0,0,' ID=1226\n')
  Lightpost_92 : Subsystem  Tag=WorldObjectSubSystem  InitFcn=UniqueId = '2311';
      mask Display: image(imread('Lightpost.jpg'));color('white');text(0,0,' ID=1227\n')
  Lightpost_93 : Subsystem  Tag=WorldObjectSubSystem  InitFcn=UniqueId = '2326';
      mask Display: image(imread('Lightpost.jpg'));color('white');text(0,0,' ID=1240\n')
  Lightpost_94 : Subsystem  Tag=WorldObjectSubSystem  InitFcn=UniqueId = '2327';
      mask Display: image(imread('Lightpost.jpg'));color('white');text(0,0,' ID=1241\n')
  Lightpost_95 : Subsystem  Tag=WorldObjectSubSystem  InitFcn=UniqueId = '2342';
      mask Display: image(imread('Lightpost.jpg'));color('white');text(0,0,' ID=1254\n')
  Lightpost_96 : Subsystem  Tag=WorldObjectSubSystem  InitFcn=UniqueId = '2343';
      mask Display: image(imread('Lightpost.jpg'));color('white');text(0,0,' ID=1255\n')
  Lightpost_97 : Subsystem  Tag=WorldObjectSubSystem  InitFcn=UniqueId = '2358';
      mask Display: image(imread('Lightpost.jpg'));color('white');text(0,0,' ID=1268\n')
  Lightpost_98 : Subsystem  Tag=WorldObjectSubSystem  InitFcn=UniqueId = '2359';
      mask Display: image(imread('Lightpost.jpg'));color('white');text(0,0,' ID=1269\n')
  Lightpost_99 : Subsystem  Tag=WorldObjectSubSystem  InitFcn=UniqueId = '2374';
      mask Display: image(imread('Lightpost.jpg'));color('white');text(0,0,' ID=1282\n')
  Mazda_RX8_1 : Subsystem  Tag=WorldObjectSubSystem  InitFcn=UniqueId = '1863';
      mask Display: image(imread('Mazda_RX8.jpg'));color('white');text(0,0,' ID=959\n')
  Mazda_RX8_2 : Subsystem  Tag=WorldObjectSubSystem  InitFcn=UniqueId = '1929';
      mask Display: image(imread('Mazda_RX8.jpg'));color('white');text(0,0,' ID=992\n')
  Mazda_RX8_3 : Subsystem  Tag=WorldObjectSubSystem  InitFcn=UniqueId = '1973';
      mask Display: image(imread('Mazda_RX8.jpg'));color('white');text(0,0,' ID=1009\n')
  Nissan_Cabstar_1 : Subsystem  Tag=WorldObjectSubSystem  InitFcn=UniqueId = '1579';
      mask Display: image(imread('Nissan_Cabstar.jpg'));color('white');text(0,0,' ID=483\n')
  SELF_Actros_1860_1 : Subsystem  Tag=SELF  Priority=3  mask(PreScan Source Block: SELF){PortName=SELF_Actros_1860_1, PortIndex=24, PortWidth=8, TimeStep=1/100}
      mask prompts: PortName "Auto-Assigned: Port Name [-]"; PortIndex "Auto-Assigned: Port Index [-]"; PortWidth "Auto-Assigned: Port Dimensions"; TimeStep "Auto-Assigned: Upload Period [s]"
      mask help:
      | The SELF port shows (state) information of the actor itself. The outputs are position in the experiment's axis system and the GPS coordinates, and the object's absolute velocity and compass heading.
      |
      | For more information, please click Help.
      settings: DeleteFcn=PreScanBlockDeleteFcn;
      mask Help: eval('openHelp(462)')
      mask Display: image(imread('GPS.png'))
  SELF_Actros_1860_2 : Subsystem  Tag=SELF  Priority=3  mask(PreScan Source Block: SELF){PortName=SELF_Actros_1860_2, PortIndex=40, PortWidth=8, TimeStep=1/100}
      mask prompts: PortName "Auto-Assigned: Port Name [-]"; PortIndex "Auto-Assigned: Port Index [-]"; PortWidth "Auto-Assigned: Port Dimensions"; TimeStep "Auto-Assigned: Upload Period [s]"
      mask help:
      | The SELF port shows (state) information of the actor itself. The outputs are position in the experiment's axis system and the GPS coordinates, and the object's absolute velocity and compass heading.
      |
      | For more information, please click Help.
      settings: DeleteFcn=PreScanBlockDeleteFcn;
      mask Help: eval('openHelp(462)')
      mask Display: image(imread('GPS.png'))
  SELF_Actros_2541_1 : Subsystem  Tag=SELF  Priority=3  mask(PreScan Source Block: SELF){PortName=SELF_Actros_2541_1, PortIndex=56, PortWidth=8, TimeStep=1/100}
      mask prompts: PortName "Auto-Assigned: Port Name [-]"; PortIndex "Auto-Assigned: Port Index [-]"; PortWidth "Auto-Assigned: Port Dimensions"; TimeStep "Auto-Assigned: Upload Period [s]"
      mask help:
      | The SELF port shows (state) information of the actor itself. The outputs are position in the experiment's axis system and the GPS coordinates, and the object's absolute velocity and compass heading.
      |
      | For more information, please click Help.
      settings: DeleteFcn=PreScanBlockDeleteFcn;
      mask Help: eval('openHelp(462)')
      mask Display: image(imread('GPS.png'))
  SELF_Actros_2541_2 : Subsystem  Tag=SELF  Priority=3  mask(PreScan Source Block: SELF){PortName=SELF_Actros_2541_2, PortIndex=192, PortWidth=8, TimeStep=1/100}
      mask prompts: PortName "Auto-Assigned: Port Name [-]"; PortIndex "Auto-Assigned: Port Index [-]"; PortWidth "Auto-Assigned: Port Dimensions"; TimeStep "Auto-Assigned: Upload Period [s]"
      mask help:
      | The SELF port shows (state) information of the actor itself. The outputs are position in the experiment's axis system and the GPS coordinates, and the object's absolute velocity and compass heading.
      |
      | For more information, please click Help.
      settings: DeleteFcn=PreScanBlockDeleteFcn;
      mask Help: eval('openHelp(462)')
      mask Display: image(imread('GPS.png'))
  SELF_Audi_A8_1 : Subsystem  Tag=SELF  Priority=3  mask(PreScan Source Block: SELF){PortName=SELF_Audi_A8_1, PortIndex=0, PortWidth=8, TimeStep=1/100}
      mask prompts: PortName "Auto-Assigned: Port Name [-]"; PortIndex "Auto-Assigned: Port Index [-]"; PortWidth "Auto-Assigned: Port Dimensions"; TimeStep "Auto-Assigned: Upload Period [s]"
      mask help:
      | The SELF port shows (state) information of the actor itself. The outputs are position in the experiment's axis system and the GPS coordinates, and the object's absolute velocity and compass heading.
      |
      | For more information, please click Help.